simulink model "CLRC_exp_D"  (R2023a)
settings: Solver=FixedStepDiscrete  StartTime=0.0  StopTime=inf  FixedStep=0.001  RelTol=1e-3  EnableMultiTasking=on
PreLoadFcn:
  | set_param(gcs, 'RTWSystemTargetFile', 'franka_emika_panda.tlc');
  | demos_common_configs();
values from neighbouring files (symbols this model uses):
  alpha = 3    [Panda_run.m]
  B = 4.44*I    [Panda_run.m]
  GammaWa = 0.2*eye(69)    [Panda_run.m]
  kapa1 = 1    [Panda_run.m]
  kapa2 = 1    [Panda_run.m]
  Kc = diag([20,20,20,20,8,8,5])    [Panda_run.m]
  Kp = kp*I    [Panda_run.m]
  Lambda = 10*eye(7)    [Panda_run.m]
  q0 = traj.qd.Data(:,:,1)    [Panda_run.m]
  taud = 25    [Panda_run.m]
  Ts = 0.001    [Panda_run.m]
undefined symbols (not defined in the file or in its folder): b1, b2, b3, DiscIntNeverNeededParam
libraries: franka_simulink_library x4
settings Model: SimulationMode=external  ExtModeTrigDuration=10000  Creator=vasi_ko  LastModifiedBy=stt
settings SolverCC: AlgebraicLoopSolver=TrustRegion
settings DataIOCC: LimitDataPoints=on  SaveFormat=Array  ReturnWorkspaceOutputs=off
settings OptimizationCC: DefaultParameterBehavior=Inlined  EnhancedBackFolding=on  PassReuseOutputArgsThreshold=0  OptimizeBlockOrder=speed  DifferentSizesBufferReuse=on  OptimizationCustomize=off
settings DebuggingCC: SolverPrmCheckMsg=warning  ParameterTunabilityLossMsg=error  UnconnectedInputMsg=warning  UnconnectedOutputMsg=warning  UnconnectedLineMsg=warning  SFUnexpectedBacktrackingDiag=warning  SFNoUnconditionalDefaultTransitionDiag=warning  SFExecutionAtInitializationDiag=none  ArithmeticOperatorsInVariantConditions=warning
settings HardwareCC: ProdBitPerLong=64  ProdHWDeviceType=Intel->x86-64 (Linux 64)
settings ModelReferenceCC: EnableRefExpFcnMdlSchedulingChecks=on  PropagateSignalLabelsOutOfModel=off
settings SFSimCC: SimUseLocalCustomCode=off  MATLABDynamicMemAlloc=off  CustomCodeUndefinedFunction=UseInterfaceOnly  SimTargetLang=C++
settings RTWCC: SystemTargetFile=franka_emika_panda.tlc  RTWVerbose=off  RTWUseLocalCustomCode=off  Toolchain=Franka Simulink | gmake (64-bit linux)  BuildConfiguration=Faster Runs  TargetLang=C++  DLArmComputeVersion=19.05
settings CodeAppCC: ForceParamTrailComments=on  OperatorAnnotations=on
settings STFCustomTargetCC: ModelReferenceCompliant=off  ConcurrentExecutionCompliant=off  MATLABClassNameForMDSCustomization=Simulink.SoftwareTarget.TargetSpecificTriggerConfigurationBase  UseToolchainInfoCompliant=off  SystemTargetFile=franka_emika_panda.tlc
settings ConfigComp: CovMetricSettings=dw  CovSaveCumulativeToWorkspaceVar=on  CovSaveSingleToWorkspaceVar=on  CovExternalEMLEnable=off  CovSFcnEnable=off

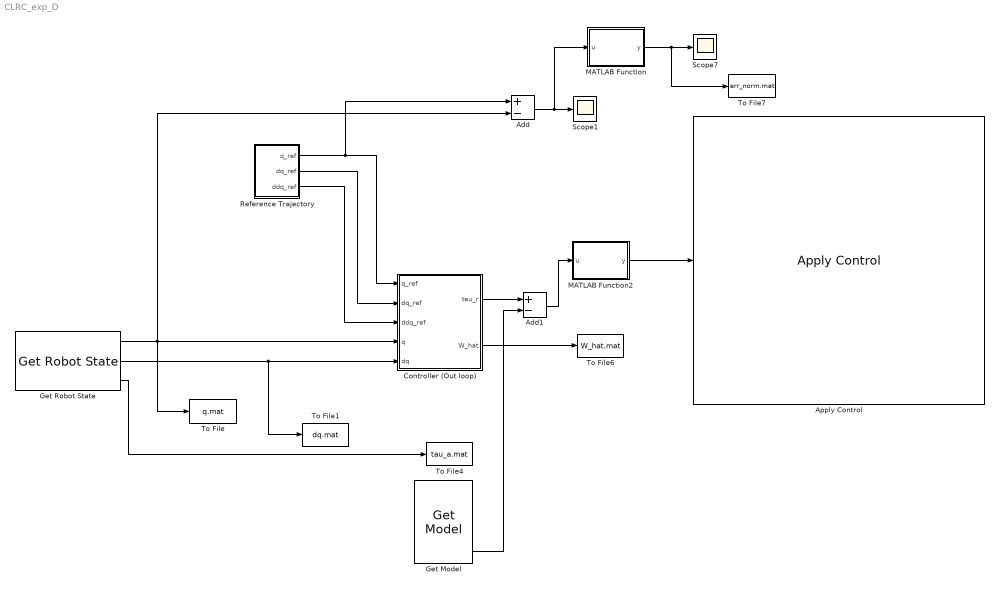
== system CLRC_exp_D ==
  Add : Sum  Inputs=+-
  Add1 : Sum  Inputs=+-
  Apply Control : franka_simulink_library/Apply Control  robot_ip=robot_ip  gripper_attached=off  control_mode=Torque Control  controller_choice=Joint Impedance Controller  elbow_control=on  collision_thresholds=collision_behavior  joint_impedance=joint_impedance  cartesian_impedance=cartesian_impedance  load_inertia=load_inertia  EE_T_K=EE_T_K  init_joint_configuration_req=on  init_joint_configuration=q0'
  Controller (Out loop) : Subsystem
  Get Model : franka_simulink_library/Get Model  robot_ip=robot_ip
  Get Robot State : franka_simulink_library/Get Robot State  robot_ip=robot_ip  signals_requested='q dq tau_J'
  MATLAB Function : MATLAB Function  TreatAsAtomicUnit=on
  MATLAB Function2 : MATLAB Function  TreatAsAtomicUnit=on
  Reference Trajectory : Subsystem
  Scope1 : Scope
  Scope7 : Scope
  To File : ToFile  Filename=q.mat  MatrixName=q
  To File1 : ToFile  Filename=dq.mat  MatrixName=dq
  To File4 : ToFile  Filename=tau_a.mat  MatrixName=tau_a
  To File6 : ToFile  Filename=W_hat.mat  MatrixName=W_hat
  To File7 : ToFile  Filename=err_norm.mat  MatrixName=err_norm
  wires:
  Get Robot State/2 -> To File1/1, Controller (Out loop)/5(dq)
  Add1/1 -> MATLAB Function2/1(u)
  Add/1 -> MATLAB Function/1(u), Scope1/1
  MATLAB Function2/1(y) -> Apply Control/1
  Reference Trajectory/3(ddq_ref) -> Controller (Out loop)/3(ddq_ref)
  Reference Trajectory/2(dq_ref) -> Controller (Out loop)/2(dq_ref)
  Get Model/6 -> Add1/2
  MATLAB Function/1(y) -> To File7/1, Scope7/1
  Controller (Out loop)/2(W_hat) -> To File6/1
  Get Robot State/3 -> To File4/1
  Controller (Out loop)/1(tau_r) -> Add1/1
  Get Robot State/1 -> Add/2, To File/1, Controller (Out loop)/4(q)
  Reference Trajectory/1(q_ref) -> Controller (Out loop)/1(q_ref), Add/1

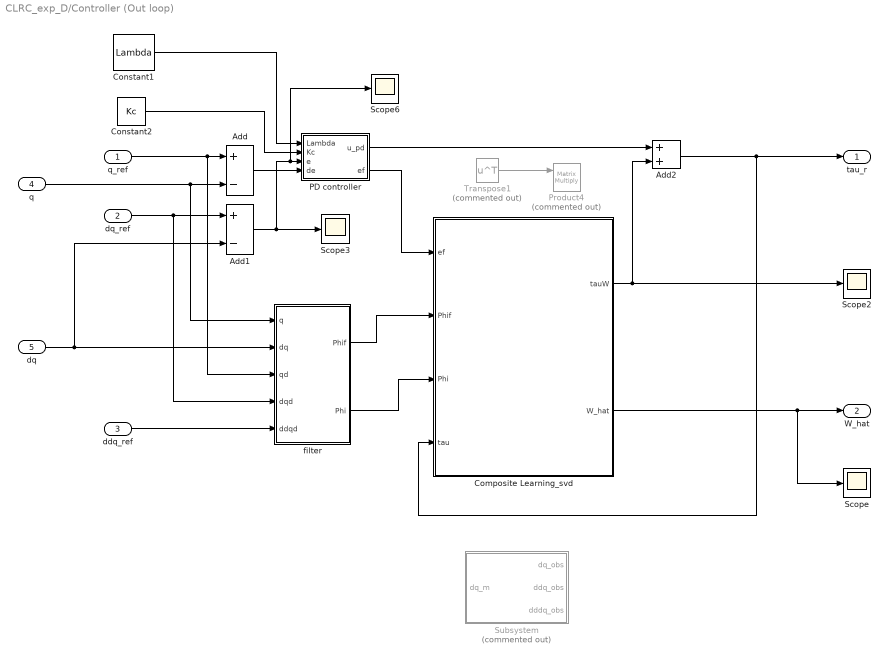
== system CLRC_exp_D/Controller (Out loop) ==
  q_ref : Inport
  dq_ref : Inport  Port=2
  ddq_ref : Inport  Port=3
  q : Inport  Port=4
  dq : Inport  Port=5
  Add : Sum  Inputs=+-
  Add1 : Sum  Inputs=+-
  Add2 : Sum
  Composite Learning_svd : Subsystem
  Constant1 : Constant  Value=Lambda
  Constant2 : Constant  Value=Kc
  PD controller : MATLAB Function  TreatAsAtomicUnit=on
  Product4 : Product  Commented=on  Multiplication=Matrix(*)
  Scope{,2..3,6} (4 alike) : Scope
  Subsystem : Subsystem  Commented=on
  Transpose1 : Math  Commented=on  Operator=transpose
  filter : Subsystem
  tau_r : Outport
  W_hat : Outport  Port=2
  wires:
  q_ref/1 -> filter/3(qd), Add/1
  dq_ref/1 -> filter/4(dqd), Add1/1
  Add/1 -> Scope6/1, PD controller/3(e)
  PD controller/1(u_pd) -> Add2/1
  Constant2/1 -> PD controller/2(Kc)
  Constant1/1 -> PD controller/1(Lambda)
  Add1/1 -> PD controller/4(de), Scope3/1
  ddq_ref/1 -> filter/5(ddqd)
  filter/1(Phif) -> Composite Learning_svd/2(Phif)
  PD controller/2(ef) -> Composite Learning_svd/1(ef)
  Composite Learning_svd/2(W_hat) -> Scope/1, W_hat/1
  filter/2(Phi) -> Composite Learning_svd/3(Phi)
  Composite Learning_svd/1(tauW) -> Add2/2, Scope2/1
  Transpose1/1 -> Product4/1
  Add2/1 -> Composite Learning_svd/4(tau), tau_r/1
  q/1 -> filter/1(q), Add/2
  dq/1 -> Add1/2, filter/2(dq)

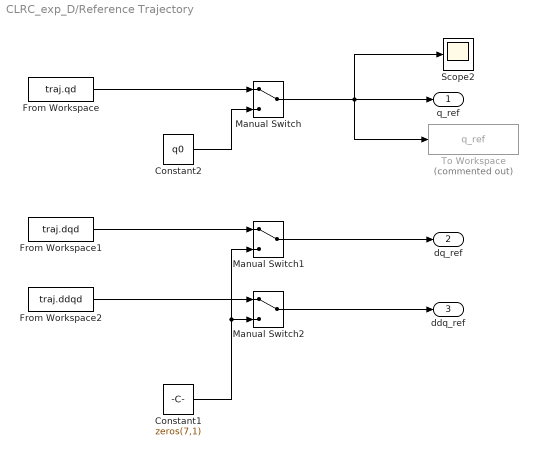
== system CLRC_exp_D/Reference Trajectory ==
  Constant1 : Constant  Value=zeros(7,1)
  Constant2 : Constant  Value=q0
  From Workspace : FromWorkspace  VariableName=traj.qd
  From Workspace1 : FromWorkspace  VariableName=traj.dqd
  From Workspace2 : FromWorkspace  VariableName=traj.ddqd
  Manual Switch : ManualSwitch
  Manual Switch1 : ManualSwitch
  Manual Switch2 : ManualSwitch
  Scope2 : Scope
  To Workspace : ToWorkspace  Commented=on  VariableName=q_ref  SaveFormat=Timeseries  SampleTime=-1
  q_ref : Outport
  dq_ref : Outport  Port=2
  ddq_ref : Outport  Port=3
  wires:
  Constant2/1 -> Manual Switch/2
  Manual Switch/1 -> To Workspace/1, Scope2/1, q_ref/1
  Manual Switch1/1 -> dq_ref/1
  Manual Switch2/1 -> ddq_ref/1
  Constant1/1 -> Manual Switch1/2, Manual Switch2/2
  From Workspace/1 -> Manual Switch/1
  From Workspace1/1 -> Manual Switch1/1
  From Workspace2/1 -> Manual Switch2/1

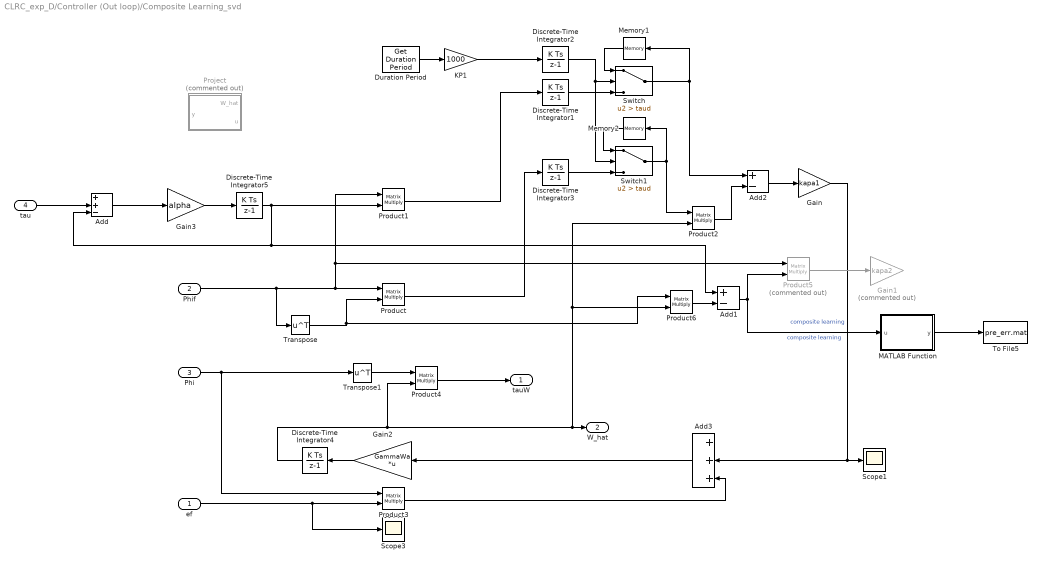
== system CLRC_exp_D/Controller (Out loop)/Composite Learning_svd ==
  ef : Inport
  Phif : Inport  Port=2
  Phi : Inport  Port=3
  tau : Inport  Port=4
  Add : Sum  Inputs=++-
  Add1 : Sum  Inputs=+-
  Add2 : Sum  Inputs=+-
  Add3 : Sum  Inputs=+++
  Discrete-Time Integrator{1..5} (5 alike) : DiscreteIntegrator  SampleTime=-1  ICPrevOutput=DiscIntNeverNeededParam  ICPrevScaledInput=DiscIntNeverNeededParam
  Duration Period : franka_simulink_library/Get Duration Period  robot_ip=robot_ip
  Gain : Gain  Gain=kapa1
  Gain1 : Gain  Commented=on  Gain=kapa2
  Gain2 : Gain  Gain=GammaWa  Multiplication=Matrix(K*u)
  Gain3 : Gain  Gain=alpha
  KP1 : Gain  Gain=1000
  MATLAB Function : MATLAB Function  TreatAsAtomicUnit=on
  Memory1 : Memory
  Memory2 : Memory
  Product{,1..4,6} (6 alike) : Product  Multiplication=Matrix(*)
  Product5 : Product  Commented=on  Multiplication=Matrix(*)
  Project : MATLAB Function  Commented=on  TreatAsAtomicUnit=on
  Scope1 : Scope
  Scope3 : Scope
  Switch : Switch  Criteria=u2 > Threshold  Threshold=taud  SaturateOnIntegerOverflow=off  InputSameDT=off
  Switch1 : Switch  Criteria=u2 > Threshold  Threshold=taud  SaturateOnIntegerOverflow=off  InputSameDT=off
  To File5 : ToFile  Filename=pre_err.mat  MatrixName=pre_err
  Transpose : Math  Operator=transpose
  Transpose1 : Math  Operator=transpose
  tauW : Outport
  W_hat : Outport  Port=2
  wires:
  Transpose/1 -> Product6/1, Product/2
  ef/1 -> Scope3/1, Product3/2
  Phi/1 -> Transpose1/1, Product3/1
  Transpose1/1 -> Product4/1
  Phif/1 -> Transpose/1, Product1/1, Product5/1, Product/1
  Gain/1 -> Add3/2, Scope1/1  "composite learning"
  Gain2/1 -> Discrete-Time Integrator4/1
  Add3/1 -> Gain2/1
  Add2/1 -> Gain/1
  Product4/1 -> tauW/1
  Product3/1 -> Add3/3
  Discrete-Time Integrator5/1 -> Product1/2, Add1/1, Add/3
  Gain3/1 -> Discrete-Time Integrator5/1
  Add/1 -> Gain3/1
  tau/1 -> Add/2
  Product1/1 -> Discrete-Time Integrator1/1
  Switch1/1 -> Memory2/1, Product2/1
  Product2/1 -> Add2/2
  Switch/1 -> Add2/1, Memory1/1
  Memory1/1 -> Switch/1
  Discrete-Time Integrator3/1 -> Switch1/3
  Discrete-Time Integrator1/1 -> Switch/3
  Discrete-Time Integrator2/1 -> Switch1/2, Switch/2
  Memory2/1 -> Switch1/1
  Discrete-Time Integrator4/1 -> Product4/2, Product6/2, Product2/2, W_hat/1
  KP1/1 -> Discrete-Time Integrator2/1
  Duration Period/1 -> KP1/1
  Product5/1 -> Gain1/1
  MATLAB Function/1(y) -> To File5/1
  Product/1 -> Discrete-Time Integrator3/1
  Product6/1 -> Add1/2
  Add1/1 -> Product5/2, MATLAB Function/1(u)  "composite learning"

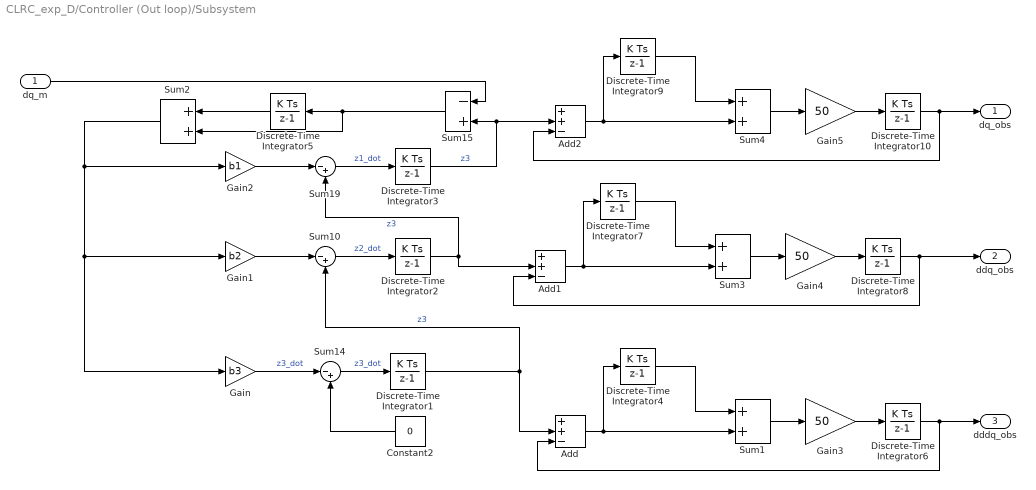
== system CLRC_exp_D/Controller (Out loop)/Subsystem ==
  dq_m : Inport
  Add : Sum  Inputs=++-
  Add1 : Sum  Inputs=++-
  Add2 : Sum  Inputs=++-
  Constant2 : Constant  Value=0
  Discrete-Time Integrator{1..10} (10 alike) : DiscreteIntegrator  SampleTime=-1  ICPrevOutput=DiscIntNeverNeededParam  ICPrevScaledInput=DiscIntNeverNeededParam
  Gain : Gain  Gain=b3
  Gain1 : Gain  Gain=b2
  Gain2 : Gain  Gain=b1
  Gain3 : Gain  Gain=50
  Gain4 : Gain  Gain=50
  Gain5 : Gain  Gain=50
  Sum{1..4} (4 alike) : Sum
  Sum10 : Sum  Inputs=|-+
  Sum14 : Sum  Inputs=|-+
  Sum15 : Sum  Inputs=-+
  Sum19 : Sum  Inputs=|-+
  dq_obs : Outport
  ddq_obs : Outport  Port=2
  dddq_obs : Outport  Port=3
  wires:
  Gain2/1 -> Sum19/1
  Gain4/1 -> Discrete-Time Integrator8/1
  Discrete-Time Integrator5/1 -> Sum2/1
  Discrete-Time Integrator7/1 -> Sum3/1
  Sum4/1 -> Gain5/1
  Sum1/1 -> Gain3/1
  Discrete-Time Integrator3/1 -> Add2/2, Sum15/2  "z3"
  Gain3/1 -> Discrete-Time Integrator6/1
  Discrete-Time Integrator8/1 -> ddq_obs/1, Add1/3
  Discrete-Time Integrator4/1 -> Sum1/1
  Gain1/1 -> Sum10/1
  Constant2/1 -> Sum14/2
  Add2/1 -> Discrete-Time Integrator9/1, Sum4/2
  Discrete-Time Integrator10/1 -> dq_obs/1, Add2/3
  Discrete-Time Integrator9/1 -> Sum4/1
  Sum15/1 -> Discrete-Time Integrator5/1, Sum2/2
  Sum3/1 -> Gain4/1
  Gain5/1 -> Discrete-Time Integrator10/1
  Sum10/1 -> Discrete-Time Integrator2/1  "z2_dot"
  Sum14/1 -> Discrete-Time Integrator1/1  "z3_dot"
  Discrete-Time Integrator1/1 -> Add/2, Sum10/2  "z3"
  Sum2/1 -> Gain2/1, Gain/1, Gain1/1
  Gain/1 -> Sum14/1  "z3_dot"
  dq_m/1 -> Sum15/1
  Add1/1 -> Discrete-Time Integrator7/1, Sum3/2
  Sum19/1 -> Discrete-Time Integrator3/1  "z1_dot"
  Discrete-Time Integrator6/1 -> dddq_obs/1, Add/3
  Add/1 -> Discrete-Time Integrator4/1, Sum1/2
  Discrete-Time Integrator2/1 -> Sum19/2, Add1/2  "z3"

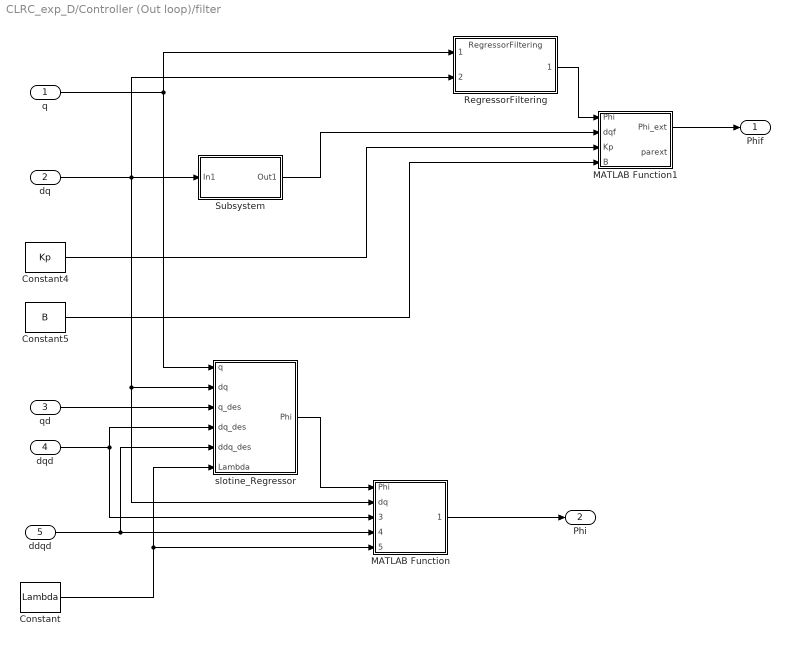
== system CLRC_exp_D/Controller (Out loop)/filter ==
  q : Inport
  dq : Inport  Port=2
  qd : Inport  Port=3
  dqd : Inport  Port=4
  ddqd : Inport  Port=5
  Constant : Constant  Value=Lambda
  Constant4 : Constant  Value=Kp
  Constant5 : Constant  Value=B
  MATLAB Function : MATLAB Function  TreatAsAtomicUnit=on
  MATLAB Function1 : MATLAB Function  TreatAsAtomicUnit=on
  RegressorFiltering : ModelReference  ModelNameDialog=RegressorFiltering  ModelReferenceVersion=9.2  CopyOfModelProtected=on
  Subsystem : Subsystem
  slotine_Regressor : MATLAB Function  TreatAsAtomicUnit=on
  Phif : Outport
  Phi : Outport  Port=2
  wires:
  qd/1 -> slotine_Regressor/3(q_des)
  dqd/1 -> MATLAB Function/3(dq_des), slotine_Regressor/4(dq_des)
  ddqd/1 -> slotine_Regressor/5(ddq_des), MATLAB Function/4(ddq_des)
  Constant/1 -> slotine_Regressor/6(Lambda), MATLAB Function/5(Lambda)
  Constant4/1 -> MATLAB Function1/3(Kp)
  Constant5/1 -> MATLAB Function1/4(B)
  slotine_Regressor/1(Phi) -> MATLAB Function/1(Phi)
  q/1 -> slotine_Regressor/1(q), RegressorFiltering/1
  Subsystem/1(Out1) -> MATLAB Function1/2(dqf)
  dq/1 -> slotine_Regressor/2(dq), MATLAB Function/2(dq), RegressorFiltering/2, Subsystem/1(In1)
  MATLAB Function/1(Phi_ext) -> Phi/1
  MATLAB Function1/1(Phi_ext) -> Phif/1
  RegressorFiltering/1 -> MATLAB Function1/1(Phi)

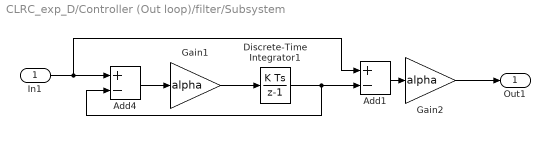
== system CLRC_exp_D/Controller (Out loop)/filter/Subsystem ==
  In1 : Inport
  Add1 : Sum  Inputs=+-
  Add4 : Sum  Inputs=+-
  Discrete-Time Integrator1 : DiscreteIntegrator  SampleTime=Ts  ICPrevOutput=DiscIntNeverNeededParam  ICPrevScaledInput=DiscIntNeverNeededParam
  Gain1 : Gain  Gain=alpha
  Gain2 : Gain  Gain=alpha
  Out1 : Outport
  wires:
  Add1/1 -> Gain2/1
  Gain1/1 -> Discrete-Time Integrator1/1
  Add4/1 -> Gain1/1
  Discrete-Time Integrator1/1 -> Add1/2, Add4/2
  Gain2/1 -> Out1/1
  In1/1 -> Add1/1, Add4/1

== matlab function CLRC_exp_D/Controller (Out loop)/PD controller ==
  data: Lambda input; Kc input; e input; u_pd output; de input; ef output
function [u_pd,ef]= fcn(Lambda,Kc,e,de)

ef=de+Lambda*e;

u_pd = Kc*ef;

== matlab function CLRC_exp_D/Controller (Out loop)/filter/slotine_Regressor ==
  data: q input; Phi output; dq input; q_des input; dq_des input; ddq_des input; Lambda input
function Phi = fcn(q,dq,q_des,dq_des,ddq_des,Lambda)
e1=q-q_des;
de1=dq-dq_des;
dqr=dq_des-Lambda*e1;
ddqr=ddq_des-Lambda*de1;

Phi=Base_Slotine_Panda(q,dq,dqr,ddqr);

== matlab function CLRC_exp_D/MATLAB Function2 ==
  data: u input; y output
function y = fcn(u)

y = u';

== matlab function CLRC_exp_D/MATLAB Function ==
  data: u input; y output
function y = fcn(u)

y = norm(u);

== matlab function CLRC_exp_D/Controller (Out loop)/Composite Learning_svd/MATLAB Function ==
  data: u input; y output
function y = fcn(u)

y = norm(u);

== matlab function CLRC_exp_D/Controller (Out loop)/Composite Learning_svd/Project ==
  data: W_hat input; u input; y output
function y = fcn(W_hat,u)
cw=10;

% if norm(W_hat)<cw
%     y=u;
% elseif abs(norm(W_hat)-cw)<0.001 && W_hat'*u<=0
%     y=u;
% else
%     y=u-W_hat*W_hat'*u/norm(W_hat)^2;
% end

y=u;

== matlab function CLRC_exp_D/Controller (Out loop)/filter/MATLAB Function ==
  data: Phi input; dq input; Phi_ext output; dq_des input; ddq_des input; Lambda input
function Phi_ext = fcn(Phi,dq,dq_des,ddq_des,Lambda)
de1=dq-dq_des;
ddqr=ddq_des-Lambda*de1;
Ma=[zeros(5,2),diag(ddqr(3:7))];
Phi_ext=[Phi;Ma];

== matlab function CLRC_exp_D/Controller (Out loop)/filter/MATLAB Function1 ==
  data: Phi input; dqf input; Phi_ext output; Kp input; B input; parext output
function [Phi_ext,parext]= fcn(Phi,dqf,Kp,B)
I=eye(7);
Ma=[zeros(5,2),diag(dqf(3:7))];
Phi_ext=[Phi;Ma];
p=diag((I+Kp)\B,0);
parext=p(3:7);
